simulink model "ClimaRateLimMgr"  (R2022b) (continued)
== system ClimaRateLimMgr == (continued)
  ClimaCfgForActrModVrnt1_ClimaCfgForActrInd1/1 -> ClimaRateLimMgr_Runnable_sys/10(ClimaCfgForActrModVrnt1_ClimaCfgForActrInd1_read)
  ClimaCfgForActrModVrnt1_ClimaCfgForActrModVrnt1/1 -> ClimaRateLimMgr_Runnable_sys/11(ClimaCfgForActrModVrnt1_ClimaCfgForActrModVrnt1_read)
  ClimaCfgForActrModVrnt1_ClimaCfgForActrModVrntCen/1 -> ClimaRateLimMgr_Runnable_sys/12(ClimaCfgForActrModVrnt1_ClimaCfgForActrModVrntCen_read)
  ClimaCfgForAmntOfSeatRow1_ClimaCfgForAmntOfSeatRow1/1 -> ClimaRateLimMgr_Runnable_sys/13(ClimaCfgForAmntOfSeatRow1_ClimaCfgForAmntOfSeatRow1_read)
  ClimaCfgForBlowrInRowSecVentSidePrsnt1_ClimaCfgForBlowrInRowSecVentSidePrsnt1/1 -> ClimaRateLimMgr_Runnable_sys/14(ClimaCfgForBlowrInRowSecVentSidePrsnt1_ClimaCfgForBlowrInRowSecVentSidePrsnt1_read)
  ClimaCfgForCarTyp1_ClimaCfgForCarTyp1/1 -> ClimaRateLimMgr_Runnable_sys/15(ClimaCfgForCarTyp1_ClimaCfgForCarTyp1_read)
  ClimaCfgForRowSecVentCentrVrnt1_ClimaCfgForRowSecVentCentrVrnt1/1 -> ClimaRateLimMgr_Runnable_sys/16(ClimaCfgForRowSecVentCentrVrnt1_ClimaCfgForRowSecVentCentrVrnt1_read)
  ClimaCfgForRowSecVentSidePrsnt1_ClimaCfgForRowSecVentSidePrsnt1/1 -> ClimaRateLimMgr_Runnable_sys/17(ClimaCfgForRowSecVentSidePrsnt1_ClimaCfgForRowSecVentSidePrsnt1_read)
  ClimaCtrlActvNgcc_ClimaCtrlActv/1 -> ClimaRateLimMgr_Runnable_sys/18(ClimaCtrlActvNgcc_ClimaCtrlActv_read)
  ClimaCtrlForEngStbActv_ClimaCtrlForEngStbActv/1 -> ClimaRateLimMgr_Runnable_sys/19(ClimaCtrlForEngStbActv_ClimaCtrlForEngStbActv_read)
  ClimaFlowPredCorrdForManModForDefrstAtStaby_ClimaFlowPredCorrdForManModForDefrstAtStaby/1 -> ClimaRateLimMgr_Runnable_sys/20(ClimaFlowPredCorrdForManModForDefrstAtStaby_ClimaFlowPredCorrdForManModForDefrstAtStaby_read)
  ClimaFlowPredCorrdForManModForDefrstNoLim_ClimaFlowPredCorrdForManModForDefrstNoLim/1 -> ClimaRateLimMgr_Runnable_sys/21(ClimaFlowPredCorrdForManModForDefrstNoLim_ClimaFlowPredCorrdForManModForDefrstNoLim_read)
  ClimaFlowPredCorrdForManModForRowFirstAtFlrAtStaby_ClimaFlowPredCorrdForManModForRowFirstAtFlrAtStaby/1 -> ClimaRateLimMgr_Runnable_sys/22(ClimaFlowPredCorrdForManModForRowFirstAtFlrAtStaby_ClimaFlowPredCorrdForManModForRowFirstAtFlrAtStaby_read)
  ClimaFlowPredCorrdForManModForRowFirstAtFlrNoLim_ClimaFlowPredCorrdForManModForRowFirstAtFlrNoLim/1 -> ClimaRateLimMgr_Runnable_sys/23(ClimaFlowPredCorrdForManModForRowFirstAtFlrNoLim_ClimaFlowPredCorrdForManModForRowFirstAtFlrNoLim_read)
  ClimaFlowPredCorrdForManModForRowFirstAtVentAtStaby_ClimaFlowPredCorrdForManModForRowFirstAtVentAtStaby/1 -> ClimaRateLimMgr_Runnable_sys/24(ClimaFlowPredCorrdForManModForRowFirstAtVentAtStaby_ClimaFlowPredCorrdForManModForRowFirstAtVentAtStaby_read)
  ClimaFlowPredCorrdForManModForRowFirstAtVentNoLim_ClimaFlowPredCorrdForManModForRowFirstAtVentNoLim/1 -> ClimaRateLimMgr_Runnable_sys/25(ClimaFlowPredCorrdForManModForRowFirstAtVentNoLim_ClimaFlowPredCorrdForManModForRowFirstAtVentNoLim_read)
  ClimaFlowPredCorrdForManModForRowSecAtFlrAtStaby_ClimaFlowPredCorrdForManModForRowSecAtFlrAtStaby/1 -> ClimaRateLimMgr_Runnable_sys/26(ClimaFlowPredCorrdForManModForRowSecAtFlrAtStaby_ClimaFlowPredCorrdForManModForRowSecAtFlrAtStaby_read)
  ClimaFlowPredCorrdForManModForRowSecAtFlrNoLim_ClimaFlowPredCorrdForManModForRowSecAtFlrNoLim/1 -> ClimaRateLimMgr_Runnable_sys/27(ClimaFlowPredCorrdForManModForRowSecAtFlrNoLim_ClimaFlowPredCorrdForManModForRowSecAtFlrNoLim_read)
  ClimaFlowPredCorrdForManModForRowSecAtVentAtStaby_ClimaFlowPredCorrdForManModForRowSecAtVentAtStaby/1 -> ClimaRateLimMgr_Runnable_sys/28(ClimaFlowPredCorrdForManModForRowSecAtVentAtStaby_ClimaFlowPredCorrdForManModForRowSecAtVentAtStaby_read)
  ClimaFlowPredCorrdForManModForRowSecAtVentNoLim_ClimaFlowPredCorrdForManModForRowSecAtVentNoLim/1 -> ClimaRateLimMgr_Runnable_sys/29(ClimaFlowPredCorrdForManModForRowSecAtVentNoLim_ClimaFlowPredCorrdForManModForRowSecAtVentNoLim_read)
  ClimaFlowReqCorrdForManModForDefrst_ClimaFlowReqCorrdForManModForDefrst/1 -> ClimaRateLimMgr_Runnable_sys/30(ClimaFlowReqCorrdForManModForDefrst_ClimaFlowReqCorrdForManModForDefrst_read)
  ClimaFlowReqCorrdForManModForDefrst_ClimaFlowReqCorrdForManModForDefrstLe/1 -> ClimaRateLimMgr_Runnable_sys/31(ClimaFlowReqCorrdForManModForDefrst_ClimaFlowReqCorrdForManModForDefrstLe_read)
  ClimaFlowReqCorrdForManModForDefrst_ClimaFlowReqCorrdForManModForDefrstRi/1 -> ClimaRateLimMgr_Runnable_sys/32(ClimaFlowReqCorrdForManModForDefrst_ClimaFlowReqCorrdForManModForDefrstRi_read)
  ClimaFlowReqCorrdForManModForRowFirstAtFlr_ClimaFlowReqCorrdForManModForRowFirstAtFlr/1 -> ClimaRateLimMgr_Runnable_sys/33(ClimaFlowReqCorrdForManModForRowFirstAtFlr_ClimaFlowReqCorrdForManModForRowFirstAtFlr_read)
  ClimaFlowReqCorrdForManModForRowFirstAtFlr_ClimaFlowReqCorrdForManModForRowFirstAtFlrLe/1 -> ClimaRateLimMgr_Runnable_sys/34(ClimaFlowReqCorrdForManModForRowFirstAtFlr_ClimaFlowReqCorrdForManModForRowFirstAtFlrLe_read)
  ClimaFlowReqCorrdForManModForRowFirstAtFlr_ClimaFlowReqCorrdForManModForRowFirstAtFlrRi/1 -> ClimaRateLimMgr_Runnable_sys/35(ClimaFlowReqCorrdForManModForRowFirstAtFlr_ClimaFlowReqCorrdForManModForRowFirstAtFlrRi_read)
  ClimaFlowReqCorrdForManModForRowFirstAtVent_ClimaFlowReqCorrdForManModForRowFirstAtVent/1 -> ClimaRateLimMgr_Runnable_sys/36(ClimaFlowReqCorrdForManModForRowFirstAtVent_ClimaFlowReqCorrdForManModForRowFirstAtVent_read)
  ClimaFlowReqCorrdForManModForRowFirstAtVent_ClimaFlowReqCorrdForManModForRowFirstAtVentLe/1 -> ClimaRateLimMgr_Runnable_sys/37(ClimaFlowReqCorrdForManModForRowFirstAtVent_ClimaFlowReqCorrdForManModForRowFirstAtVentLe_read)
  ClimaFlowReqCorrdForManModForRowFirstAtVent_ClimaFlowReqCorrdForManModForRowFirstAtVentLeMid/1 -> ClimaRateLimMgr_Runnable_sys/38(ClimaFlowReqCorrdForManModForRowFirstAtVent_ClimaFlowReqCorrdForManModForRowFirstAtVentLeMid_read)
  ClimaFlowReqCorrdForManModForRowFirstAtVent_ClimaFlowReqCorrdForManModForRowFirstAtVentLeSide/1 -> ClimaRateLimMgr_Runnable_sys/39(ClimaFlowReqCorrdForManModForRowFirstAtVent_ClimaFlowReqCorrdForManModForRowFirstAtVentLeSide_read)
  ClimaFlowReqCorrdForManModForRowFirstAtVent_ClimaFlowReqCorrdForManModForRowFirstAtVentRi/1 -> ClimaRateLimMgr_Runnable_sys/40(ClimaFlowReqCorrdForManModForRowFirstAtVent_ClimaFlowReqCorrdForManModForRowFirstAtVentRi_read)
  ClimaFlowReqCorrdForManModForRowFirstAtVent_ClimaFlowReqCorrdForManModForRowFirstAtVentRiMid/1 -> ClimaRateLimMgr_Runnable_sys/41(ClimaFlowReqCorrdForManModForRowFirstAtVent_ClimaFlowReqCorrdForManModForRowFirstAtVentRiMid_read)
  ClimaFlowReqCorrdForManModForRowFirstAtVent_ClimaFlowReqCorrdForManModForRowFirstAtVentRiSide/1 -> ClimaRateLimMgr_Runnable_sys/42(ClimaFlowReqCorrdForManModForRowFirstAtVent_ClimaFlowReqCorrdForManModForRowFirstAtVentRiSide_read)
  ClimaFlowReqCorrdForManModForRowSecAtFlr_ClimaFlowReqCorrdForManModForRowSecAtFlr/1 -> ClimaRateLimMgr_Runnable_sys/43(ClimaFlowReqCorrdForManModForRowSecAtFlr_ClimaFlowReqCorrdForManModForRowSecAtFlr_read)
  ClimaFlowReqCorrdForManModForRowSecAtFlr_ClimaFlowReqCorrdForManModForRowSecAtFlrLe/1 -> ClimaRateLimMgr_Runnable_sys/44(ClimaFlowReqCorrdForManModForRowSecAtFlr_ClimaFlowReqCorrdForManModForRowSecAtFlrLe_read)
  ClimaFlowReqCorrdForManModForRowSecAtFlr_ClimaFlowReqCorrdForManModForRowSecAtFlrRi/1 -> ClimaRateLimMgr_Runnable_sys/45(ClimaFlowReqCorrdForManModForRowSecAtFlr_ClimaFlowReqCorrdForManModForRowSecAtFlrRi_read)
  ClimaFlowReqCorrdForManModForRowSecAtVent_ClimaFlowReqCorrdForManModForRowSecAtVent/1 -> ClimaRateLimMgr_Runnable_sys/46(ClimaFlowReqCorrdForManModForRowSecAtVent_ClimaFlowReqCorrdForManModForRowSecAtVent_read)
  ClimaFlowReqCorrdForManModForRowSecAtVent_ClimaFlowReqCorrdForManModForRowSecAtVentLe/1 -> ClimaRateLimMgr_Runnable_sys/47(ClimaFlowReqCorrdForManModForRowSecAtVent_ClimaFlowReqCorrdForManModForRowSecAtVentLe_read)
  ClimaFlowReqCorrdForManModForRowSecAtVent_ClimaFlowReqCorrdForManModForRowSecAtVentRi/1 -> ClimaRateLimMgr_Runnable_sys/48(ClimaFlowReqCorrdForManModForRowSecAtVent_ClimaFlowReqCorrdForManModForRowSecAtVentRi_read)
  ClimaStStrtUp_ClimaStStrtUp/1 -> ClimaRateLimMgr_Runnable_sys/49(ClimaStStrtUp_ClimaStStrtUp_read)
  CnvincModActv_CnvincModActv/1 -> ClimaRateLimMgr_Runnable_sys/50(CnvincModActv_CnvincModActv_read)
  CoolgReqForDispActv_CoolgReqForDispActv/1 -> ClimaRateLimMgr_Runnable_sys/51(CoolgReqForDispActv_CoolgReqForDispActv_read)
  FacForRiLeDifForClimaCmftFlowForRowSec_FacForRiLeDifForClimaCmftFlowForRowSec/1 -> ClimaRateLimMgr_Runnable_sys/52(FacForRiLeDifForClimaCmftFlowForRowSec_FacForRiLeDifForClimaCmftFlowForRowSec_read)
  FanLvlMaxActv_FanLvlMaxActv/1 -> ClimaRateLimMgr_Runnable_sys/53(FanLvlMaxActv_FanLvlMaxActv_read)
  FlowRednAtPwrAllwdLo_FlowRednAtPwrAllwdLo/1 -> ClimaRateLimMgr_Runnable_sys/54(FlowRednAtPwrAllwdLo_FlowRednAtPwrAllwdLo_read)
  FlowRednAtPwrLvlLo_FlowRednAtPwrLvlLo/1 -> ClimaRateLimMgr_Runnable_sys/55(FlowRednAtPwrLvlLo_FlowRednAtPwrLvlLo_read)
  FltModForAmbTActv_FltModForAmbTActv/1 -> ClimaRateLimMgr_Runnable_sys/56(FltModForAmbTActv_FltModForAmbTActv_read)
  FogFlashPrevnActv_FogFlashPrevnActv/1 -> ClimaRateLimMgr_Runnable_sys/57(FogFlashPrevnActv_FogFlashPrevnActv_read)
  HmiCmptmtAirDistbnFrntForClima_HmiCmptmtAirDistbnFrntForClima/1 -> ClimaRateLimMgr_Runnable_sys/58(HmiCmptmtAirDistbnFrntForClima_HmiCmptmtAirDistbnFrntForClima_read)
  HmiCmptmtAirDistbnFrntForClima_HmiCmptmtAirDistbnFrntRiForClima/1 -> ClimaRateLimMgr_Runnable_sys/59(HmiCmptmtAirDistbnFrntForClima_HmiCmptmtAirDistbnFrntRiForClima_read)
  HmiCmptmtAirDistbnFrntForClima_HmiCmptmtAirDistbnReForClima/1 -> ClimaRateLimMgr_Runnable_sys/60(HmiCmptmtAirDistbnFrntForClima_HmiCmptmtAirDistbnReForClima_read)
  HmiCmptmtAirDistbnFrntForClima_HmiCmptmtAirDistbnReRiForClima/1 -> ClimaRateLimMgr_Runnable_sys/61(HmiCmptmtAirDistbnFrntForClima_HmiCmptmtAirDistbnReRiForClima_read)
  HmiCmptmtTSpForClima_HmiCmptmtTSpForClima/1 -> ClimaRateLimMgr_Runnable_sys/62(HmiCmptmtTSpForClima_HmiCmptmtTSpForClima_read)
  HmiDefrstMaxReqForClima_HmiDefrstMaxReqForClima/1 -> ClimaRateLimMgr_Runnable_sys/63(HmiDefrstMaxReqForClima_HmiDefrstMaxReqForClima_read)
  HmiHvacFanLvlFrntForClima_HmiHvacFanLvlFrntForClima/1 -> ClimaRateLimMgr_Runnable_sys/64(HmiHvacFanLvlFrntForClima_HmiHvacFanLvlFrntForClima_read)
  HmiHvacFanLvlFrntForClima_HmiHvacFanLvlFrntRiForClima/1 -> ClimaRateLimMgr_Runnable_sys/65(HmiHvacFanLvlFrntForClima_HmiHvacFanLvlFrntRiForClima_read)
  HmiMaxACReqForClima_HmiMaxACReqForClima/1 -> ClimaRateLimMgr_Runnable_sys/66(HmiMaxACReqForClima_HmiMaxACReqForClima_read)
  HvacAirMFlowEstimd_Ngcc_HvacAirMFlowEstimd/1 -> ClimaRateLimMgr_Runnable_sys/67(HvacAirMFlowEstimd_Ngcc_HvacAirMFlowEstimd_read)
  HvacFlowFrntActForDefrst_HvacFlowFrntActForDefrst/1 -> ClimaRateLimMgr_Runnable_sys/68(HvacFlowFrntActForDefrst_HvacFlowFrntActForDefrst_read)
  HvacFlowFrntActForRowFirstAtFlr_HvacFlowFrntActForRowFirstAtFlr/1 -> ClimaRateLimMgr_Runnable_sys/69(HvacFlowFrntActForRowFirstAtFlr_HvacFlowFrntActForRowFirstAtFlr_read)
  HvacFlowFrntActForRowFirstAtFlr_HvacFlowFrntActForRowFirstAtFlrLe/1 -> ClimaRateLimMgr_Runnable_sys/70(HvacFlowFrntActForRowFirstAtFlr_HvacFlowFrntActForRowFirstAtFlrLe_read)
  HvacFlowFrntActForRowFirstAtFlr_HvacFlowFrntActForRowFirstAtFlrRi/1 -> ClimaRateLimMgr_Runnable_sys/71(HvacFlowFrntActForRowFirstAtFlr_HvacFlowFrntActForRowFirstAtFlrRi_read)
  HvacFlowFrntActForRowFirstAtVent_HvacFlowFrntActForRowFirstAtVent/1 -> ClimaRateLimMgr_Runnable_sys/72(HvacFlowFrntActForRowFirstAtVent_HvacFlowFrntActForRowFirstAtVent_read)
  HvacFlowFrntActForRowFirstAtVent_HvacFlowFrntActForRowFirstAtVentLe/1 -> ClimaRateLimMgr_Runnable_sys/73(HvacFlowFrntActForRowFirstAtVent_HvacFlowFrntActForRowFirstAtVentLe_read)
  HvacFlowFrntActForRowFirstAtVent_HvacFlowFrntActForRowFirstAtVentRi/1 -> ClimaRateLimMgr_Runnable_sys/74(HvacFlowFrntActForRowFirstAtVent_HvacFlowFrntActForRowFirstAtVentRi_read)
  HvacFlowFrntActForRowFirstAtVent_HvacFlowFrntActForRowFirstAtVentSideLe/1 -> ClimaRateLimMgr_Runnable_sys/75(HvacFlowFrntActForRowFirstAtVent_HvacFlowFrntActForRowFirstAtVentSideLe_read)
  HvacFlowFrntActForRowFirstAtVent_HvacFlowFrntActForRowFirstAtVentSideRi/1 -> ClimaRateLimMgr_Runnable_sys/76(HvacFlowFrntActForRowFirstAtVent_HvacFlowFrntActForRowFirstAtVentSideRi_read)
  HvacFlowFrntActForRowSecAtFlr_HvacFlowFrntActForRowSecAtFlr/1 -> ClimaRateLimMgr_Runnable_sys/77(HvacFlowFrntActForRowSecAtFlr_HvacFlowFrntActForRowSecAtFlr_read)
  HvacFlowFrntActForRowSecAtFlr_HvacFlowFrntActForRowSecAtFlrLe/1 -> ClimaRateLimMgr_Runnable_sys/78(HvacFlowFrntActForRowSecAtFlr_HvacFlowFrntActForRowSecAtFlrLe_read)
  HvacFlowFrntActForRowSecAtFlr_HvacFlowFrntActForRowSecAtFlrRi/1 -> ClimaRateLimMgr_Runnable_sys/79(HvacFlowFrntActForRowSecAtFlr_HvacFlowFrntActForRowSecAtFlrRi_read)
  HvacFlowFrntActForRowSecAtVent_HvacFlowFrntActForRowSecAtVent/1 -> ClimaRateLimMgr_Runnable_sys/80(HvacFlowFrntActForRowSecAtVent_HvacFlowFrntActForRowSecAtVent_read)
  HvacFlowFrntActForRowSecAtVent_HvacFlowFrntActForRowSecAtVentLe/1 -> ClimaRateLimMgr_Runnable_sys/81(HvacFlowFrntActForRowSecAtVent_HvacFlowFrntActForRowSecAtVentLe_read)
  HvacFlowFrntActForRowSecAtVent_HvacFlowFrntActForRowSecAtVentRi/1 -> ClimaRateLimMgr_Runnable_sys/82(HvacFlowFrntActForRowSecAtVent_HvacFlowFrntActForRowSecAtVentRi_read)
  HvacFlowFrntRednEnad_HvacFlowFrntRednEnad/1 -> ClimaRateLimMgr_Runnable_sys/83(HvacFlowFrntRednEnad_HvacFlowFrntRednEnad_read)
  HvacFlrLeakReqForRowFirst_HvacFlrLeakReqForRowFirst/1 -> ClimaRateLimMgr_Runnable_sys/84(HvacFlrLeakReqForRowFirst_HvacFlrLeakReqForRowFirst_read)
  HvacFlrLeakReqForRowFirstPredForStaby_HvacFlrLeakReqForRowFirstPredForStaby/1 -> ClimaRateLimMgr_Runnable_sys/85(HvacFlrLeakReqForRowFirstPredForStaby_HvacFlrLeakReqForRowFirstPredForStaby_read)
  HvacFlrLeakReqForRowSec_HvacFlrLeakReqForRowSec/1 -> ClimaRateLimMgr_Runnable_sys/86(HvacFlrLeakReqForRowSec_HvacFlrLeakReqForRowSec_read)
  HvacFlrLeakReqForRowSecPredForStaby_HvacFlrLeakReqForRowSecPredForStaby/1 -> ClimaRateLimMgr_Runnable_sys/87(HvacFlrLeakReqForRowSecPredForStaby_HvacFlrLeakReqForRowSecPredForStaby_read)
  InteCleanUnpleSmell_Ngcc_InteCleanUnpleSmell/1 -> ClimaRateLimMgr_Runnable_sys/88(InteCleanUnpleSmell_Ngcc_InteCleanUnpleSmell_read)
  ManModeForRowFirstLeForModelDial_ManModeForRowFirstLeForModelDial/1 -> ClimaRateLimMgr_Runnable_sys/89(ManModeForRowFirstLeForModelDial_ManModeForRowFirstLeForModelDial_read)
  ManModeForRowFirstRiForModelDial_ManModeForRowFirstRiForModelDial/1 -> ClimaRateLimMgr_Runnable_sys/90(ManModeForRowFirstRiForModelDial_ManModeForRowFirstRiForModelDial_read)
  ManModeForRowSecLeForModelDial_ManModeForRowSecLeForModelDial/1 -> ClimaRateLimMgr_Runnable_sys/91(ManModeForRowSecLeForModelDial_ManModeForRowSecLeForModelDial_read)
  TModCtrlAtRowSec_TModCtrlAtRowSec/1 -> ClimaRateLimMgr_Runnable_sys/92(TModCtrlAtRowSec_TModCtrlAtRowSec_read)
  TModCtrlAtRowSec_TModCtrlAtRowSecLe/1 -> ClimaRateLimMgr_Runnable_sys/93(TModCtrlAtRowSec_TModCtrlAtRowSecLe_read)
  TModCtrlAtRowSec_TModCtrlAtRowSecRi/1 -> ClimaRateLimMgr_Runnable_sys/94(TModCtrlAtRowSec_TModCtrlAtRowSecRi_read)
  ActvnTiForClimaCtrlRe_ActvnTiForClimaCtrlRe/1 -> ClimaRateLimMgr_Runnable_sys/95(ActvnTiForClimaCtrlRe_ActvnTiForClimaCtrlRe_read)
  AirTFromHeatgEstimdLimd_AirTFromHeatgEstimdLimd/1 -> ClimaRateLimMgr_Runnable_sys/96(AirTFromHeatgEstimdLimd_AirTFromHeatgEstimdLimd_read)
  AirTFromHeatgEstimdLimdRe_AirTFromHeatgEstimdLimdRe/1 -> ClimaRateLimMgr_Runnable_sys/97(AirTFromHeatgEstimdLimdRe_AirTFromHeatgEstimdLimdRe_read)
  AntiHotFlowToFaceActvFrnt_AntiHotFlowToFaceActvFrnt/1 -> ClimaRateLimMgr_Runnable_sys/98(AntiHotFlowToFaceActvFrnt_AntiHotFlowToFaceActvFrnt_read)
  AntiHotFlowToFaceActvRe_AntiHotFlowToFaceActvRe/1 -> ClimaRateLimMgr_Runnable_sys/99(AntiHotFlowToFaceActvRe_AntiHotFlowToFaceActvRe_read)
  AutDemstOperSts_AutDemstOperSts/1 -> ClimaRateLimMgr_Runnable_sys/100(AutDemstOperSts_AutDemstOperSts_read)
  ClimaAtRowSecActvNgcc_ClimaAtRowSecActv/1 -> ClimaRateLimMgr_Runnable_sys/101(ClimaAtRowSecActvNgcc_ClimaAtRowSecActv_read)
  ClimaAtRowSecActvNgcc_ClimaAtRowSecActvLe/1 -> ClimaRateLimMgr_Runnable_sys/102(ClimaAtRowSecActvNgcc_ClimaAtRowSecActvLe_read)
  ClimaAtRowSecActvNgcc_ClimaAtRowSecActvRi/1 -> ClimaRateLimMgr_Runnable_sys/103(ClimaAtRowSecActvNgcc_ClimaAtRowSecActvRi_read)
  ClimaCfgHvacFrntForRowSecForActrTLe_ClimaCfgHvacFrntForRowSecForActrTLe/1 -> ClimaRateLimMgr_Runnable_sys/104(ClimaCfgHvacFrntForRowSecForActrTLe_ClimaCfgHvacFrntForRowSecForActrTLe_read)
  ClimaCfgHvacFrntForRowSecForActrTRi_ClimaCfgHvacFrntForRowSecForActrTRi/1 -> ClimaRateLimMgr_Runnable_sys/105(ClimaCfgHvacFrntForRowSecForActrTRi_ClimaCfgHvacFrntForRowSecForActrTRi_read)
  ClimaCfgReqVersionReplace_ClimaCfgReqVersionReplace/1 -> ClimaRateLimMgr_Runnable_sys/106(ClimaCfgReqVersionReplace_ClimaCfgReqVersionReplace_read)
  ClimaStStrtUpRe_ClimaStStrtUpRe/1 -> ClimaRateLimMgr_Runnable_sys/107(ClimaStStrtUpRe_ClimaStStrtUpRe_read)
  EcoModForClimaActv_EcoModForClimaActv/1 -> ClimaRateLimMgr_Runnable_sys/108(EcoModForClimaActv_EcoModForClimaActv_read)
  FlowDecDurgSpdIdleActvFlg_FlowDecDurgSpdIdleActvFlg/1 -> ClimaRateLimMgr_Runnable_sys/109(FlowDecDurgSpdIdleActvFlg_FlowDecDurgSpdIdleActvFlg_read)
  FlowDecDurgSpdIdleActvFlgRe_FlowDecDurgSpdIdleActvFlgRe/1 -> ClimaRateLimMgr_Runnable_sys/110(FlowDecDurgSpdIdleActvFlgRe_FlowDecDurgSpdIdleActvFlgRe_read)
  FlowLimActv_FlowLimActv/1 -> ClimaRateLimMgr_Runnable_sys/111(FlowLimActv_FlowLimActv_read)
  FlowLimActvRe_FlowLimActvRe/1 -> ClimaRateLimMgr_Runnable_sys/112(FlowLimActvRe_FlowLimActvRe_read)
  FlowRednForEngStby_FlowRednForEngStby/1 -> ClimaRateLimMgr_Runnable_sys/113(FlowRednForEngStby_FlowRednForEngStby_read)
  FrntClimaManModCmftStsNgcc_FrntClimaManModCmftStsNgcc/1 -> ClimaRateLimMgr_Runnable_sys/114(FrntClimaManModCmftStsNgcc_FrntClimaManModCmftStsNgcc_read)
  HmiHvacFanLvlReForClima_HmiHvacFanLvlReForClima/1 -> ClimaRateLimMgr_Runnable_sys/115(HmiHvacFanLvlReForClima_HmiHvacFanLvlReForClima_read)
  HmiHvacFanLvlReForClima_HmiHvacFanLvlReRiForClima/1 -> ClimaRateLimMgr_Runnable_sys/116(HmiHvacFanLvlReForClima_HmiHvacFanLvlReRiForClima_read)
  HvacFlowRednEnadRe_HvacFlowRednEnadRe/1 -> ClimaRateLimMgr_Runnable_sys/117(HvacFlowRednEnadRe_HvacFlowRednEnadRe_read)
  LowrWtrTActvn_LowrWtrTActvn/1 -> ClimaRateLimMgr_Runnable_sys/118(LowrWtrTActvn_LowrWtrTActvn_read)
  ManModeForRowThrdForModelDial_ManModeForRowThrdForModelDial/1 -> ClimaRateLimMgr_Runnable_sys/119(ManModeForRowThrdForModelDial_ManModeForRowThrdForModelDial_read)
  PrkgClimaOnReq_PrkgClimaOnReq/1 -> ClimaRateLimMgr_Runnable_sys/120(PrkgClimaOnReq_PrkgClimaOnReq_read)
  PrkgClimaOnReqRe_PrkgClimaOnReqRe/1 -> ClimaRateLimMgr_Runnable_sys/121(PrkgClimaOnReqRe_PrkgClimaOnReqRe_read)
  RuranModeSetAirFlowActv_RuranModeSetAirFlowActv/1 -> ClimaRateLimMgr_Runnable_sys/122(RuranModeSetAirFlowActv_RuranModeSetAirFlowActv_read)
  ClimaRateLimMgr_Runnable_sys/1(ClimaFlowReqForDefrstWithFltMod_ClimaFlowReqForDefrstWithFltMod_write) -> ClimaFlowReqForDefrstWithFltMod_ClimaFlowReqForDefrstWithFltMod/1
  ClimaRateLimMgr_Runnable_sys/2(ClimaFlowReqForRowFirstAtFlrWithFltMod_ClimaFlowReqForRowFirstAtFlrWithFltMod_write) -> ClimaFlowReqForRowFirstAtFlrWithFltMod_ClimaFlowReqForRowFirstAtFlrWithFltMod/1
  ClimaRateLimMgr_Runnable_sys/3(ClimaFlowReqForRowFirstAtVentWithFltMod_ClimaFlowReqForRowFirstAtVentWithFltMod_write) -> ClimaFlowReqForRowFirstAtVentWithFltMod_ClimaFlowReqForRowFirstAtVentWithFltMod/1
  ClimaRateLimMgr_Runnable_sys/4(ClimaFlowReqForRowSecAtFlrWithFltMod_ClimaFlowReqForRowSecAtFlrWithFltMod_write) -> ClimaFlowReqForRowSecAtFlrWithFltMod_ClimaFlowReqForRowSecAtFlrWithFltMod/1
  ClimaRateLimMgr_Runnable_sys/5(ClimaFlowReqForRowSecAtVentWithFltMod_ClimaFlowReqForRowSecAtVentWithFltMod_write) -> ClimaFlowReqForRowSecAtVentWithFltMod_ClimaFlowReqForRowSecAtVentWithFltMod/1
  ClimaRateLimMgr_Runnable_sys/6(AirFlowAddlForDispCoolg_AirFlowAddlForDispCoolg_write) -> AirFlowAddlForDispCoolg_AirFlowAddlForDispCoolg/1
  ClimaRateLimMgr_Runnable_sys/7(ClimaRateVCCAirFlowRampTyp_ClimaRateVCCAirFlowRampTyp_write) -> ClimaRateVCCAirFlowRampTyp_ClimaRateVCCAirFlowRampTyp/1
  ClimaRateLimMgr_Runnable_sys/8(FacForRiLeDifForHvacFlowForVentRowSec_FacForRiLeDifForHvacFlowForVentRowSec_write) -> FacForRiLeDifForHvacFlowForVentRowSec_FacForRiLeDifForHvacFlowForVentRowSec/1
  ClimaRateLimMgr_Runnable_sys/9(HvacAirMFlowEstimdPredAtStabyForCoolg_HvacAirMFlowEstimdPredAtStabyForCoolg_write) -> HvacAirMFlowEstimdPredAtStabyForCoolg_HvacAirMFlowEstimdPredAtStabyForCoolg/1
  ClimaRateLimMgr_Runnable_sys/10(HvacAirMFlowVentNgcc_HvacAirMFlowVent_write) -> HvacAirMFlowVentNgcc_HvacAirMFlowVent/1
  ClimaRateLimMgr_Runnable_sys/11(HvacFlowFrntReqForDefrst_HvacFlowFrntReqForDefrst_write) -> HvacFlowFrntReqForDefrst_HvacFlowFrntReqForDefrst/1
  ClimaRateLimMgr_Runnable_sys/12(HvacFlowFrntReqForRowFirstAtFlr_HvacFlowFrntReqForRowFirstAtFlr_write) -> HvacFlowFrntReqForRowFirstAtFlr_HvacFlowFrntReqForRowFirstAtFlr/1
  ClimaRateLimMgr_Runnable_sys/13(HvacFlowFrntReqForRowFirstAtFlr_HvacFlowFrntReqForRowFirstAtFlrLe_write) -> HvacFlowFrntReqForRowFirstAtFlr_HvacFlowFrntReqForRowFirstAtFlrLe/1
  ClimaRateLimMgr_Runnable_sys/14(HvacFlowFrntReqForRowFirstAtFlr_HvacFlowFrntReqForRowFirstAtFlrRi_write) -> HvacFlowFrntReqForRowFirstAtFlr_HvacFlowFrntReqForRowFirstAtFlrRi/1
  ClimaRateLimMgr_Runnable_sys/15(HvacFlowFrntReqForRowFirstAtVent_HvacFlowFrntReqForRowFirstAtVent_write) -> HvacFlowFrntReqForRowFirstAtVent_HvacFlowFrntReqForRowFirstAtVent/1
  ClimaRateLimMgr_Runnable_sys/16(HvacFlowFrntReqForRowFirstAtVent_HvacFlowFrntReqForRowFirstAtVentLe_write) -> HvacFlowFrntReqForRowFirstAtVent_HvacFlowFrntReqForRowFirstAtVentLe/1
  ClimaRateLimMgr_Runnable_sys/17(HvacFlowFrntReqForRowFirstAtVent_HvacFlowFrntReqForRowFirstAtVentLeMid_write) -> HvacFlowFrntReqForRowFirstAtVent_HvacFlowFrntReqForRowFirstAtVentLeMid/1
  ClimaRateLimMgr_Runnable_sys/18(HvacFlowFrntReqForRowFirstAtVent_HvacFlowFrntReqForRowFirstAtVentLeSide_write) -> HvacFlowFrntReqForRowFirstAtVent_HvacFlowFrntReqForRowFirstAtVentLeSide/1
  ClimaRateLimMgr_Runnable_sys/19(HvacFlowFrntReqForRowFirstAtVent_HvacFlowFrntReqForRowFirstAtVentRi_write) -> HvacFlowFrntReqForRowFirstAtVent_HvacFlowFrntReqForRowFirstAtVentRi/1
  ClimaRateLimMgr_Runnable_sys/20(HvacFlowFrntReqForRowFirstAtVent_HvacFlowFrntReqForRowFirstAtVentRiMid_write) -> HvacFlowFrntReqForRowFirstAtVent_HvacFlowFrntReqForRowFirstAtVentRiMid/1
  ClimaRateLimMgr_Runnable_sys/21(HvacFlowFrntReqForRowFirstAtVent_HvacFlowFrntReqForRowFirstAtVentRiSide_write) -> HvacFlowFrntReqForRowFirstAtVent_HvacFlowFrntReqForRowFirstAtVentRiSide/1
  ClimaRateLimMgr_Runnable_sys/22(HvacFlowFrntReqForRowSecAtFlr_HvacFlowFrntReqForRowSecAtFlr_write) -> HvacFlowFrntReqForRowSecAtFlr_HvacFlowFrntReqForRowSecAtFlr/1
  ClimaRateLimMgr_Runnable_sys/23(HvacFlowFrntReqForRowSecAtFlr_HvacFlowFrntReqForRowSecAtFlrLe_write) -> HvacFlowFrntReqForRowSecAtFlr_HvacFlowFrntReqForRowSecAtFlrLe/1
  ClimaRateLimMgr_Runnable_sys/24(HvacFlowFrntReqForRowSecAtFlr_HvacFlowFrntReqForRowSecAtFlrRi_write) -> HvacFlowFrntReqForRowSecAtFlr_HvacFlowFrntReqForRowSecAtFlrRi/1
  ClimaRateLimMgr_Runnable_sys/25(HvacFlowFrntReqForRowSecAtVent_HvacFlowFrntReqForRowSecAtVent_write) -> HvacFlowFrntReqForRowSecAtVent_HvacFlowFrntReqForRowSecAtVent/1
  ClimaRateLimMgr_Runnable_sys/26(HvacFlowFrntReqForRowSecAtVent_HvacFlowFrntReqForRowSecAtVentLe_write) -> HvacFlowFrntReqForRowSecAtVent_HvacFlowFrntReqForRowSecAtVentLe/1
  ClimaRateLimMgr_Runnable_sys/27(HvacFlowFrntReqForRowSecAtVent_HvacFlowFrntReqForRowSecAtVentRi_write) -> HvacFlowFrntReqForRowSecAtVent_HvacFlowFrntReqForRowSecAtVentRi/1
  ClimaRateLimMgr_Runnable_sys/28(HvacFlowPredForDefrstAtStaby_HvacFlowPredForDefrstAtStaby_write) -> HvacFlowPredForDefrstAtStaby_HvacFlowPredForDefrstAtStaby/1
  ClimaRateLimMgr_Runnable_sys/29(HvacFlowPredForDefrstNoLim_HvacFlowPredForDefrstNoLim_write) -> HvacFlowPredForDefrstNoLim_HvacFlowPredForDefrstNoLim/1
  ClimaRateLimMgr_Runnable_sys/30(HvacFlowPredForRowFirstAtFlrAtStaby_HvacFlowPredForRowFirstAtFlrAtStaby_write) -> HvacFlowPredForRowFirstAtFlrAtStaby_HvacFlowPredForRowFirstAtFlrAtStaby/1
  ClimaRateLimMgr_Runnable_sys/31(HvacFlowPredForRowFirstAtFlrNoLim_HvacFlowPredForRowFirstAtFlrNoLim_write) -> HvacFlowPredForRowFirstAtFlrNoLim_HvacFlowPredForRowFirstAtFlrNoLim/1
  ClimaRateLimMgr_Runnable_sys/32(HvacFlowPredForRowFirstAtVentAtStaby_HvacFlowPredForRowFirstAtVentAtStaby_write) -> HvacFlowPredForRowFirstAtVentAtStaby_HvacFlowPredForRowFirstAtVentAtStaby/1
  ClimaRateLimMgr_Runnable_sys/33(HvacFlowPredForRowFirstAtVentNoLim_HvacFlowPredForRowFirstAtVentNoLim_write) -> HvacFlowPredForRowFirstAtVentNoLim_HvacFlowPredForRowFirstAtVentNoLim/1
  ClimaRateLimMgr_Runnable_sys/34(HvacFlowPredForRowSecAtFlrAtStaby_HvacFlowPredForRowSecAtFlrAtStaby_write) -> HvacFlowPredForRowSecAtFlrAtStaby_HvacFlowPredForRowSecAtFlrAtStaby/1
  ClimaRateLimMgr_Runnable_sys/35(HvacFlowPredForRowSecAtFlrNoLim_HvacFlowPredForRowSecAtFlrNoLim_write) -> HvacFlowPredForRowSecAtFlrNoLim_HvacFlowPredForRowSecAtFlrNoLim/1
  ClimaRateLimMgr_Runnable_sys/36(HvacFlowPredForRowSecAtVentAtStaby_HvacFlowPredForRowSecAtVentAtStaby_write) -> HvacFlowPredForRowSecAtVentAtStaby_HvacFlowPredForRowSecAtVentAtStaby/1
  ClimaRateLimMgr_Runnable_sys/37(HvacFlowPredForRowSecAtVentNoLim_HvacFlowPredForRowSecAtVentNoLim_write) -> HvacFlowPredForRowSecAtVentNoLim_HvacFlowPredForRowSecAtVentNoLim/1
  ClimaRateLimMgr_Runnable_sys/38(HvacTotFlowForCmptmt_HvacTotFlowForCmptmt_write) -> HvacTotFlowForCmptmt_HvacTotFlowForCmptmt/1
  ClimaRateLimMgr_Runnable_sys/39(HvacTotFlowPredAtStaby_HvacTotFlowPredAtStaby_write) -> HvacTotFlowPredAtStaby_HvacTotFlowPredAtStaby/1
  ClimaRateLimMgr_Runnable_sys/40(HvacTotFlowPredNoLim_HvacTotFlowPredNoLim_write) -> HvacTotFlowPredNoLim_HvacTotFlowPredNoLim/1
  ClimaRateLimMgr_Runnable/1 -> ClimaRateLimMgr_Runnable_sys/Trigger
